simulink model "AcasXuClosedLoop_all"  (Simulink 10.0)
settings: Solver=VariableStepAuto  StartTime=0.0  StopTime=20  RelTol=1e-3
libraries: neural x14040, simulink x2
(part 1 of 534)
settings Model: Creator=manzand  LastModifiedBy=manzand
settings DataIOCC: SaveFormat=Dataset  ReturnWorkspaceOutputs=on
settings OptimizationCC: LifeSpan=auto
settings DebuggingCC: UnderspecifiedInitializationDetection=Simplified  MergeDetectMultiDrivingBlocksExec=error  SFUnexpectedBacktrackingDiag=error  SFNoUnconditionalDefaultTransitionDiag=error  SFExecutionAtInitializationDiag=warning  ArithmeticOperatorsInVariantConditions=error
settings HardwareCC: ProdBitPerPointer=64  ProdBitPerSizeT=64  ProdBitPerPtrDiffT=64  ProdLargestAtomicFloat=Float  ProdIntDivRoundTo=Zero  ProdEndianess=LittleEndian  ProdWordSize=64  ProdHWDeviceType=Intel->x86-64 (Windows64)
settings ModelReferenceCC: PropagateSignalLabelsOutOfModel=on
settings SFSimCC: SimUseLocalCustomCode=on  CustomCodeUndefinedFunction=FilterOut
settings RTWCC: RTWUseLocalCustomCode=on
settings CodeAppCC: StateflowObjectComments=off
settings GRTTargetCC: TargetLangStandard=C99 (ISO)  CombineOutputUpdateFcns=on  GRTInterface=off
settings ConfigComp: CovMetricSettings=dwe  CovHtmlReporting=off  CovSaveCumulativeToWorkspaceVar=off  CovSaveSingleToWorkspaceVar=off

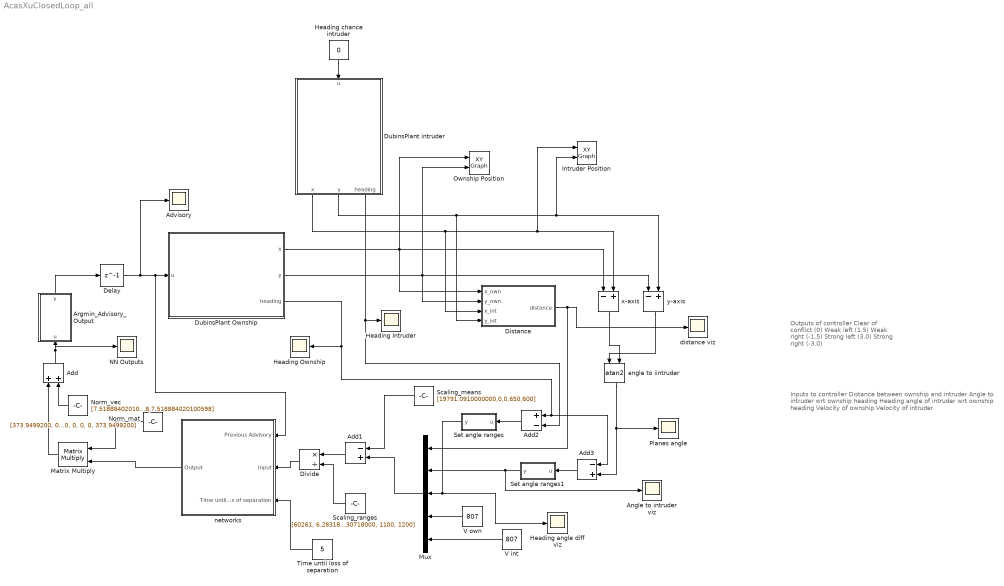
== system AcasXuClosedLoop_all ==
  note: Outputs of controller
      | Clear of conflict (0)
      | Weak left (1.5)
      | Weak right (-1.5)
      | Strong left (3.0)
      | Strong right (-3.0)
  note: Inputs to controller
      | Distance between ownship and intruder
      | Angle to intruder wrt ownship heading
      | Heading angle of intruder wrt ownship heading
      | Velocity of ownship
      | Velocity of intruder
  Add : Sum  Priority=20
  Add1 : Sum  Priority=14  Inputs=-+
  Add2 : Sum  Priority=9  Inputs=+-
  Add3 : Sum  Priority=9  Inputs=-+
  Advisory : Scope
  Angle to intruder viz : Scope
  Argmin_Advisory_Output : MATLAB Function  Priority=21  TreatAsAtomicUnit=on
  Delay : Delay  Priority=1  DelayLength=1  InitialCondition=0
  Distance : MATLAB Function  Priority=5  TreatAsAtomicUnit=on
  Divide : Product  Priority=16  Inputs=*/
  DubinsPlant Ownship : Stateflow chart  Priority=2  BlockKeywords=__PRM__SERIALIZED__DATA__:Stateflow Chart,Stateflow Diagram  TreatAsAtomicUnit=on
  DubinsPlant intruder : Stateflow chart  Priority=4  BlockKeywords=__PRM__SERIALIZED__DATA__:Stateflow Chart,Stateflow Diagram  TreatAsAtomicUnit=on
  Heading Intruder : Scope
  Heading Ownship : Scope
  Heading angle diff viz : Scope
  "Heading chance intruder " : Constant  Priority=3  Value=0
  Intruder Position : simulink/Sinks/XY Graph  xmin=-100  xmax=100  ymin=10000  ymax=14000  st=-1
  Matrix Multiply : Product  Priority=19  Multiplication=Matrix(*)
  Mux : Mux  Inputs=5
  NN Outputs : Scope
  Norm_mat : Constant  Priority=18  Value=|  VectorParams1D=off
      | [373.9499200, 0, 0, 0, 0; 0, 373.9499200, 0, 0, 0;
      | 0, 0, 373.9499200, 0, 0;
      | 0, 0, 0, 373.9499200, 0;
      | 0, 0, 0, 0, 373.9499200]
  "Norm_vec " : Constant  Value=[7.518884020100598, 7.518884020100598,7.518884020100598,7.518884020100598,7.518884020100598]
  Ownship Position : simulink/Sinks/XY Graph  xmin=-5000  xmax=600  ymin=1  ymax=23000  st=-1
  Planes angle : Scope
  Scaling_means : Constant  Priority=13  Value=[19791.0910000000,0,0,650,600]
  Scaling_ranges : Constant  Priority=15  Value=[60261, 6.28318530718000, 6.28318530718000, 1100, 1200]  VectorParams1D=off
  Set angle ranges : MATLAB Function  TreatAsAtomicUnit=on
  Set angle ranges1 : MATLAB Function  TreatAsAtomicUnit=on
  Time until loss of separation : Constant  Priority=10  Value=5
  V int : Constant  Priority=11  Value=807
  V own : Constant  Priority=10  Value=807
  angle to iintruder : Trigonometry  Priority=8  Operator=atan2  OutputSignalType=real
  distance viz : Scope
  networks : Subsystem
  x-axis : Sum  Priority=6  Inputs=-+
  y-axis : Sum  Priority=7  Inputs=-+
  wires:
  "Heading chance intruder "/1 -> DubinsPlant intruder/1(u)
  Argmin_Advisory_Output/1(y) -> Delay/1
  Scaling_ranges/1 -> Divide/2
  V int/1 -> Mux/5
  Add2/1 -> Set angle ranges/1(u)
  y-axis/1 -> angle to iintruder/1
  Add3/1 -> Set angle ranges1/1(u)
  Divide/1 -> networks/2(Input)
  Add1/1 -> Divide/1
  V own/1 -> Mux/4
  x-axis/1 -> angle to iintruder/2
  "Norm_vec "/1 -> Add/2
  Add/1 -> NN Outputs/1, Argmin_Advisory_Output/1(u)
  angle to iintruder/1 -> Planes angle/1, Add3/2
  Norm_mat/1 -> Matrix Multiply/1
  Scaling_means/1 -> Add1/1
  Distance/1(distance) -> Mux/1, distance viz/1
  DubinsPlant Ownship/3(heading) -> Heading Ownship/1, Add3/1, Add2/1
  DubinsPlant Ownship/1(x) -> Distance/1(x_own), x-axis/1, Ownship Position/1
  DubinsPlant intruder/2(y) -> Distance/4(y_int), y-axis/2, Intruder Position/2
  DubinsPlant intruder/1(x) -> Distance/3(x_int), x-axis/2, Intruder Position/1
  DubinsPlant intruder/3(heading) -> Heading Intruder/1, Add2/2
  DubinsPlant Ownship/2(y) -> Distance/2(y_own), y-axis/1, Ownship Position/2
  Delay/1 -> networks/1(Previous Advisory), DubinsPlant Ownship/1(u), Advisory/1
  networks/1(Output) -> Matrix Multiply/2
  Mux/1 -> Add1/2
  Matrix Multiply/1 -> Add/1
  Time until loss of separation/1 -> networks/3("Time until  loss of separation ")
  Set angle ranges/1(y) -> Mux/3, Heading angle diff viz/1
  Set angle ranges1/1(y) -> Angle to intruder viz/1, Mux/2

== system AcasXuClosedLoop_all/networks ==
  Previous Advisory : Inport
  Input : Inport  Port=2
  "Time until  loss of separation " : Inport  Port=3
  If : If  IfExpression=u1 == 0  ElseIfExpressions=u1 == 1, u1 == 5, u1 == 10, u1 == 20, u1 == 40, u1 == 60, u1 == 80, u1 == 100  ShowElse=off
  If Action Subsystem : Subsystem  TreatAsAtomicUnit=on
  If Action Subsystem1 : Subsystem  TreatAsAtomicUnit=on
  If Action Subsystem2 : Subsystem  TreatAsAtomicUnit=on
  If Action Subsystem3 : Subsystem  TreatAsAtomicUnit=on
  If Action Subsystem4 : Subsystem  TreatAsAtomicUnit=on
  If Action Subsystem5 : Subsystem  TreatAsAtomicUnit=on
  If Action Subsystem6 : Subsystem  TreatAsAtomicUnit=on
  If Action Subsystem7 : Subsystem  TreatAsAtomicUnit=on
  If Action Subsystem8 : Subsystem  TreatAsAtomicUnit=on
  Input{1..8} (8 alike) : InportShadow  Port=2
  Merge : Merge  Inputs=9
  Networks_m_1 : Subsystem
  Networks_m_2 : Subsystem
  Networks_m_3 : Subsystem
  Networks_m_4 : Subsystem
  Networks_m_5 : Subsystem
  Networks_m_6 : Subsystem
  Networks_m_7 : Subsystem
  Networks_m_8 : Subsystem
  Networks_m_9 : Subsystem
  Previous Advisory{1..8} (8 alike) : InportShadow
  Output : Outport
  wires:
  Merge/1 -> Output/1
  Networks_m_1/1(Out1) -> If Action Subsystem/1(In1)
  If Action Subsystem/1(Out1) -> Merge/1
  If Action Subsystem1/1(Out1) -> Merge/2
  Networks_m_2/1(Out1) -> If Action Subsystem1/1(In1)
  Networks_m_3/1(Out1) -> If Action Subsystem2/1(In1)
  If Action Subsystem2/1(Out1) -> Merge/3
  Networks_m_4/1(Out1) -> If Action Subsystem3/1(In1)
  If Action Subsystem3/1(Out1) -> Merge/4
  Networks_m_5/1(Out1) -> If Action Subsystem4/1(In1)
  If Action Subsystem4/1(Out1) -> Merge/5
  Networks_m_6/1(Out1) -> If Action Subsystem5/1(In1)
  If Action Subsystem5/1(Out1) -> Merge/6
  Networks_m_7/1(Out1) -> If Action Subsystem6/1(In1)
  If Action Subsystem6/1(Out1) -> Merge/7
  Networks_m_8/1(Out1) -> If Action Subsystem7/1(In1)
  If Action Subsystem7/1(Out1) -> Merge/8
  Networks_m_9/1(Out1) -> If Action Subsystem8/1(In1)
  If Action Subsystem8/1(Out1) -> Merge/9
  "Time until  loss of separation "/1 -> If/1
  Previous Advisory/1 -> Networks_m_1/2(Prev. advisory)
  If/1 -> If Action Subsystem/Action
  Input/1 -> Networks_m_1/1(Input)
  If/2 -> If Action Subsystem1/Action
  If/3 -> If Action Subsystem2/Action
  If/4 -> If Action Subsystem3/Action
  If/5 -> If Action Subsystem4/Action
  If/6 -> If Action Subsystem5/Action
  If/7 -> If Action Subsystem6/Action
  If/8 -> If Action Subsystem7/Action
  If/9 -> If Action Subsystem8/Action
  Input1/1 -> Networks_m_2/1(Input)
  Input2/1 -> Networks_m_3/1(Input)
  Input3/1 -> Networks_m_4/1(Input)
  Input4/1 -> Networks_m_5/1(Input)
  Input5/1 -> Networks_m_6/1(Input)
  Input6/1 -> Networks_m_7/1(Input)
  Input7/1 -> Networks_m_8/1(Input)
  Input8/1 -> Networks_m_9/1(Input)
  Previous Advisory1/1 -> Networks_m_2/2(Prev. advisory)
  Previous Advisory2/1 -> Networks_m_3/2(Prev. advisory)
  Previous Advisory3/1 -> Networks_m_4/2(Prev. advisory)
  Previous Advisory4/1 -> Networks_m_5/2(Prev. advisory)
  Previous Advisory5/1 -> Networks_m_6/2(Prev. advisory)
  Previous Advisory6/1 -> Networks_m_7/2(Prev. advisory)
  Previous Advisory7/1 -> Networks_m_8/2(Prev. advisory)
  Previous Advisory8/1 -> Networks_m_9/2(Prev. advisory)

== system AcasXuClosedLoop_all/networks/If Action Subsystem ==
  In1 : Inport
  Action Port : ActionPort  ActionPortLabel=if(u1 == 0)
  Out1 : Outport
  wires:
  In1/1 -> Out1/1

== system AcasXuClosedLoop_all/networks/If Action Subsystem1 ==
  In1 : Inport
  Action Port : ActionPort  ActionPortLabel=elseif(u1 == 1)
  Out1 : Outport
  wires:
  In1/1 -> Out1/1

== system AcasXuClosedLoop_all/networks/If Action Subsystem2 ==
  In1 : Inport
  Action Port : ActionPort  ActionPortLabel=elseif( u1 == 5)
  Out1 : Outport
  wires:
  In1/1 -> Out1/1

== system AcasXuClosedLoop_all/networks/If Action Subsystem3 ==
  In1 : Inport
  Action Port : ActionPort  ActionPortLabel=elseif( u1 == 10)
  Out1 : Outport
  wires:
  In1/1 -> Out1/1

== system AcasXuClosedLoop_all/networks/If Action Subsystem4 ==
  In1 : Inport
  Action Port : ActionPort  ActionPortLabel=elseif( u1 == 20)
  Out1 : Outport
  wires:
  In1/1 -> Out1/1

== system AcasXuClosedLoop_all/networks/If Action Subsystem5 ==
  In1 : Inport
  Action Port : ActionPort  ActionPortLabel=elseif( u1 == 40)
  Out1 : Outport
  wires:
  In1/1 -> Out1/1

== system AcasXuClosedLoop_all/networks/If Action Subsystem6 ==
  In1 : Inport
  Action Port : ActionPort  ActionPortLabel=elseif( u1 == 60)
  Out1 : Outport
  wires:
  In1/1 -> Out1/1

== system AcasXuClosedLoop_all/networks/If Action Subsystem7 ==
  In1 : Inport
  Action Port : ActionPort  ActionPortLabel=elseif( u1 == 80)
  Out1 : Outport
  wires:
  In1/1 -> Out1/1

== system AcasXuClosedLoop_all/networks/If Action Subsystem8 ==
  In1 : Inport
  Action Port : ActionPort  ActionPortLabel=elseif( u1 == 100)
  Out1 : Outport
  wires:
  In1/1 -> Out1/1

== system AcasXuClosedLoop_all/networks/Networks_m_1 ==
  Input : Inport
  Prev. advisory : Inport  Port=2
  AcasXu_1_1 : Subsystem
      mask help: Feed-Forward Neural Network
      mask Display: e = 0.06;c1 = [120 150 230]/255;c2 = ([120 150 230]+0)/383;c3 = ([120 150 230]+128)/383;patch([0 1 1 0],[0 0 1 1],c1);patch([0 1 1 1-e 1-e 0+e],[0 0 1 1-e 0+e 0+e],c2);patch([0 0 1 1-e 0+e 0+e],[0 1 1 1-e 1-e 0+e],c3);plot([0 1 1 0 0],[0 0 1 1 0]);fprintf('NNET');port_label('input',1,' Input');port_label('output',1,'Output ');
  AcasXu_2_1 : Subsystem
      mask help: Feed-Forward Neural Network
      mask Display: e = 0.06;c1 = [120 150 230]/255;c2 = ([120 150 230]+0)/383;c3 = ([120 150 230]+128)/383;patch([0 1 1 0],[0 0 1 1],c1);patch([0 1 1 1-e 1-e 0+e],[0 0 1 1-e 0+e 0+e],c2);patch([0 0 1 1-e 0+e 0+e],[0 1 1 1-e 1-e 0+e],c3);plot([0 1 1 0 0],[0 0 1 1 0]);fprintf('NNET');port_label('input',1,' Input');port_label('output',1,'Output ');
  AcasXu_3_1 : Subsystem
      mask help: Feed-Forward Neural Network
      mask Display: e = 0.06;c1 = [120 150 230]/255;c2 = ([120 150 230]+0)/383;c3 = ([120 150 230]+128)/383;patch([0 1 1 0],[0 0 1 1],c1);patch([0 1 1 1-e 1-e 0+e],[0 0 1 1-e 0+e 0+e],c2);patch([0 0 1 1-e 0+e 0+e],[0 1 1 1-e 1-e 0+e],c3);plot([0 1 1 0 0],[0 0 1 1 0]);fprintf('NNET');port_label('input',1,' Input');port_label('output',1,'Output ');
  AcasXu_4_1 : Subsystem
      mask help: Feed-Forward Neural Network
      mask Display: e = 0.06;c1 = [120 150 230]/255;c2 = ([120 150 230]+0)/383;c3 = ([120 150 230]+128)/383;patch([0 1 1 0],[0 0 1 1],c1);patch([0 1 1 1-e 1-e 0+e],[0 0 1 1-e 0+e 0+e],c2);patch([0 0 1 1-e 0+e 0+e],[0 1 1 1-e 1-e 0+e],c3);plot([0 1 1 0 0],[0 0 1 1 0]);fprintf('NNET');port_label('input',1,' Input');port_label('output',1,'Output ');
  AcasXu_5_1 : Subsystem
      mask help: Feed-Forward Neural Network
      mask Display: e = 0.06;c1 = [120 150 230]/255;c2 = ([120 150 230]+0)/383;c3 = ([120 150 230]+128)/383;patch([0 1 1 0],[0 0 1 1],c1);patch([0 1 1 1-e 1-e 0+e],[0 0 1 1-e 0+e 0+e],c2);patch([0 0 1 1-e 0+e 0+e],[0 1 1 1-e 1-e 0+e],c3);plot([0 1 1 0 0],[0 0 1 1 0]);fprintf('NNET');port_label('input',1,' Input');port_label('output',1,'Output ');
  If : If  IfExpression=u1 == 0  ElseIfExpressions=u1 == deg2rad(1.5), u1 == deg2rad(-1.5), u1 == deg2rad(3.0), u1 == deg2rad(-3.0)  ShowElse=off
  If Action Subsystem : Subsystem  TreatAsAtomicUnit=on
  If Action Subsystem1 : Subsystem  TreatAsAtomicUnit=on
  If Action Subsystem2 : Subsystem  TreatAsAtomicUnit=on
  If Action Subsystem3 : Subsystem  TreatAsAtomicUnit=on
  If Action Subsystem4 : Subsystem  TreatAsAtomicUnit=on
  Merge : Merge  Inputs=5
  Out1 : Outport
  wires:
  If Action Subsystem2/1(Out1) -> Merge/3
  If/1 -> If Action Subsystem/Action
  If/3 -> If Action Subsystem2/Action
  If Action Subsystem4/1(Out1) -> Merge/5
  Input/1 -> AcasXu_5_1/1(Input), AcasXu_4_1/1(Input), AcasXu_3_1/1(Input), AcasXu_1_1/1(Input), AcasXu_2_1/1(Input)
  If/2 -> If Action Subsystem1/Action
  If Action Subsystem1/1(Out1) -> Merge/2
  AcasXu_1_1/1(Output) -> If Action Subsystem/1(In1)
  Merge/1 -> Out1/1
  Prev. advisory/1 -> If/1
  If Action Subsystem3/1(Out1) -> Merge/4
  If/4 -> If Action Subsystem3/Action
  If/5 -> If Action Subsystem4/Action
  If Action Subsystem/1(Out1) -> Merge/1
  AcasXu_2_1/1(Output) -> If Action Subsystem1/1(In1)
  AcasXu_3_1/1(Output) -> If Action Subsystem2/1(In1)
  AcasXu_4_1/1(Output) -> If Action Subsystem3/1(In1)
  AcasXu_5_1/1(Output) -> If Action Subsystem4/1(In1)

== system AcasXuClosedLoop_all/networks/Networks_m_2 ==
  Input : Inport
  Prev. advisory : Inport  Port=2
  AcasXu_1_2 : Subsystem
      mask help: Feed-Forward Neural Network
      mask Display: e = 0.06;c1 = [120 150 230]/255;c2 = ([120 150 230]+0)/383;c3 = ([120 150 230]+128)/383;patch([0 1 1 0],[0 0 1 1],c1);patch([0 1 1 1-e 1-e 0+e],[0 0 1 1-e 0+e 0+e],c2);patch([0 0 1 1-e 0+e 0+e],[0 1 1 1-e 1-e 0+e],c3);plot([0 1 1 0 0],[0 0 1 1 0]);fprintf('NNET');port_label('input',1,' Input');port_label('output',1,'Output ');
  AcasXu_2_2 : Subsystem
      mask help: Feed-Forward Neural Network
      mask Display: e = 0.06;c1 = [120 150 230]/255;c2 = ([120 150 230]+0)/383;c3 = ([120 150 230]+128)/383;patch([0 1 1 0],[0 0 1 1],c1);patch([0 1 1 1-e 1-e 0+e],[0 0 1 1-e 0+e 0+e],c2);patch([0 0 1 1-e 0+e 0+e],[0 1 1 1-e 1-e 0+e],c3);plot([0 1 1 0 0],[0 0 1 1 0]);fprintf('NNET');port_label('input',1,' Input');port_label('output',1,'Output ');
  AcasXu_3_2 : Subsystem
      mask help: Feed-Forward Neural Network
      mask Display: e = 0.06;c1 = [120 150 230]/255;c2 = ([120 150 230]+0)/383;c3 = ([120 150 230]+128)/383;patch([0 1 1 0],[0 0 1 1],c1);patch([0 1 1 1-e 1-e 0+e],[0 0 1 1-e 0+e 0+e],c2);patch([0 0 1 1-e 0+e 0+e],[0 1 1 1-e 1-e 0+e],c3);plot([0 1 1 0 0],[0 0 1 1 0]);fprintf('NNET');port_label('input',1,' Input');port_label('output',1,'Output ');
  AcasXu_4_2 : Subsystem
      mask help: Feed-Forward Neural Network
      mask Display: e = 0.06;c1 = [120 150 230]/255;c2 = ([120 150 230]+0)/383;c3 = ([120 150 230]+128)/383;patch([0 1 1 0],[0 0 1 1],c1);patch([0 1 1 1-e 1-e 0+e],[0 0 1 1-e 0+e 0+e],c2);patch([0 0 1 1-e 0+e 0+e],[0 1 1 1-e 1-e 0+e],c3);plot([0 1 1 0 0],[0 0 1 1 0]);fprintf('NNET');port_label('input',1,' Input');port_label('output',1,'Output ');
  AcasXu_5_2 : Subsystem
      mask help: Feed-Forward Neural Network
      mask Display: e = 0.06;c1 = [120 150 230]/255;c2 = ([120 150 230]+0)/383;c3 = ([120 150 230]+128)/383;patch([0 1 1 0],[0 0 1 1],c1);patch([0 1 1 1-e 1-e 0+e],[0 0 1 1-e 0+e 0+e],c2);patch([0 0 1 1-e 0+e 0+e],[0 1 1 1-e 1-e 0+e],c3);plot([0 1 1 0 0],[0 0 1 1 0]);fprintf('NNET');port_label('input',1,' Input');port_label('output',1,'Output ');
  If : If  IfExpression=u1 == 0  ElseIfExpressions=u1 == deg2rad(1.5), u1 == deg2rad(-1.5), u1 == deg2rad(3.0), u1 == deg2rad(-3.0)  ShowElse=off
  If Action Subsystem : Subsystem  TreatAsAtomicUnit=on
  If Action Subsystem1 : Subsystem  TreatAsAtomicUnit=on
  If Action Subsystem2 : Subsystem  TreatAsAtomicUnit=on
  If Action Subsystem3 : Subsystem  TreatAsAtomicUnit=on
  If Action Subsystem4 : Subsystem  TreatAsAtomicUnit=on
  Merge : Merge  Inputs=5
  Out1 : Outport
  wires:
  AcasXu_5_2/1(Output) -> If Action Subsystem4/1(In1)
  AcasXu_4_2/1(Output) -> If Action Subsystem3/1(In1)
  AcasXu_3_2/1(Output) -> If Action Subsystem2/1(In1)
  AcasXu_2_2/1(Output) -> If Action Subsystem1/1(In1)
  If Action Subsystem/1(Out1) -> Merge/1
  If/5 -> If Action Subsystem4/Action
  If/4 -> If Action Subsystem3/Action
  If Action Subsystem3/1(Out1) -> Merge/4
  Prev. advisory/1 -> If/1
  Merge/1 -> Out1/1
  AcasXu_1_2/1(Output) -> If Action Subsystem/1(In1)
  If Action Subsystem1/1(Out1) -> Merge/2
  If/2 -> If Action Subsystem1/Action
  Input/1 -> AcasXu_2_2/1(Input), AcasXu_1_2/1(Input), AcasXu_3_2/1(Input), AcasXu_5_2/1(Input), AcasXu_4_2/1(Input)
  If Action Subsystem4/1(Out1) -> Merge/5
  If/3 -> If Action Subsystem2/Action
  If/1 -> If Action Subsystem/Action
  If Action Subsystem2/1(Out1) -> Merge/3

== system AcasXuClosedLoop_all/networks/Networks_m_3 ==
  Input : Inport
  Prev. advisory : Inport  Port=2
  AcasXu_1_3 : Subsystem
      mask help: Feed-Forward Neural Network
      mask Display: e = 0.06;c1 = [120 150 230]/255;c2 = ([120 150 230]+0)/383;c3 = ([120 150 230]+128)/383;patch([0 1 1 0],[0 0 1 1],c1);patch([0 1 1 1-e 1-e 0+e],[0 0 1 1-e 0+e 0+e],c2);patch([0 0 1 1-e 0+e 0+e],[0 1 1 1-e 1-e 0+e],c3);plot([0 1 1 0 0],[0 0 1 1 0]);fprintf('NNET');port_label('input',1,' Input');port_label('output',1,'Output ');
  AcasXu_2_3 : Subsystem
      mask help: Feed-Forward Neural Network
      mask Display: e = 0.06;c1 = [120 150 230]/255;c2 = ([120 150 230]+0)/383;c3 = ([120 150 230]+128)/383;patch([0 1 1 0],[0 0 1 1],c1);patch([0 1 1 1-e 1-e 0+e],[0 0 1 1-e 0+e 0+e],c2);patch([0 0 1 1-e 0+e 0+e],[0 1 1 1-e 1-e 0+e],c3);plot([0 1 1 0 0],[0 0 1 1 0]);fprintf('NNET');port_label('input',1,' Input');port_label('output',1,'Output ');
  AcasXu_3_3 : Subsystem
      mask help: Feed-Forward Neural Network
      mask Display: e = 0.06;c1 = [120 150 230]/255;c2 = ([120 150 230]+0)/383;c3 = ([120 150 230]+128)/383;patch([0 1 1 0],[0 0 1 1],c1);patch([0 1 1 1-e 1-e 0+e],[0 0 1 1-e 0+e 0+e],c2);patch([0 0 1 1-e 0+e 0+e],[0 1 1 1-e 1-e 0+e],c3);plot([0 1 1 0 0],[0 0 1 1 0]);fprintf('NNET');port_label('input',1,' Input');port_label('output',1,'Output ');
  AcasXu_4_3 : Subsystem
      mask help: Feed-Forward Neural Network
      mask Display: e = 0.06;c1 = [120 150 230]/255;c2 = ([120 150 230]+0)/383;c3 = ([120 150 230]+128)/383;patch([0 1 1 0],[0 0 1 1],c1);patch([0 1 1 1-e 1-e 0+e],[0 0 1 1-e 0+e 0+e],c2);patch([0 0 1 1-e 0+e 0+e],[0 1 1 1-e 1-e 0+e],c3);plot([0 1 1 0 0],[0 0 1 1 0]);fprintf('NNET');port_label('input',1,' Input');port_label('output',1,'Output ');
  AcasXu_5_3 : Subsystem
      mask help: Feed-Forward Neural Network
      mask Display: e = 0.06;c1 = [120 150 230]/255;c2 = ([120 150 230]+0)/383;c3 = ([120 150 230]+128)/383;patch([0 1 1 0],[0 0 1 1],c1);patch([0 1 1 1-e 1-e 0+e],[0 0 1 1-e 0+e 0+e],c2);patch([0 0 1 1-e 0+e 0+e],[0 1 1 1-e 1-e 0+e],c3);plot([0 1 1 0 0],[0 0 1 1 0]);fprintf('NNET');port_label('input',1,' Input');port_label('output',1,'Output ');
  If : If  IfExpression=u1 == 0  ElseIfExpressions=u1 == deg2rad(1.5), u1 == deg2rad(-1.5), u1 == deg2rad(3.0), u1 == deg2rad(-3.0)  ShowElse=off
  If Action Subsystem : Subsystem  TreatAsAtomicUnit=on
  If Action Subsystem1 : Subsystem  TreatAsAtomicUnit=on
  If Action Subsystem2 : Subsystem  TreatAsAtomicUnit=on
  If Action Subsystem3 : Subsystem  TreatAsAtomicUnit=on
  If Action Subsystem4 : Subsystem  TreatAsAtomicUnit=on
  Merge : Merge  Inputs=5
  Out1 : Outport
  wires:
  If Action Subsystem2/1(Out1) -> Merge/3
  If/1 -> If Action Subsystem/Action
  If/3 -> If Action Subsystem2/Action
  If Action Subsystem4/1(Out1) -> Merge/5
  Input/1 -> AcasXu_3_3/1(Input), AcasXu_5_3/1(Input), AcasXu_4_3/1(Input), AcasXu_2_3/1(Input), AcasXu_1_3/1(Input)
  If/2 -> If Action Subsystem1/Action
  If Action Subsystem1/1(Out1) -> Merge/2
  AcasXu_1_3/1(Output) -> If Action Subsystem/1(In1)
  Merge/1 -> Out1/1
  Prev. advisory/1 -> If/1
  If Action Subsystem3/1(Out1) -> Merge/4
  If/4 -> If Action Subsystem3/Action
  If/5 -> If Action Subsystem4/Action
  If Action Subsystem/1(Out1) -> Merge/1
  AcasXu_2_3/1(Output) -> If Action Subsystem1/1(In1)
  AcasXu_3_3/1(Output) -> If Action Subsystem2/1(In1)
  AcasXu_4_3/1(Output) -> If Action Subsystem3/1(In1)
  AcasXu_5_3/1(Output) -> If Action Subsystem4/1(In1)

== system AcasXuClosedLoop_all/networks/Networks_m_4 ==
  Input : Inport
  Prev. advisory : Inport  Port=2
  AcasXu_1_4 : Subsystem
      mask help: Feed-Forward Neural Network
      mask Display: e = 0.06;c1 = [120 150 230]/255;c2 = ([120 150 230]+0)/383;c3 = ([120 150 230]+128)/383;patch([0 1 1 0],[0 0 1 1],c1);patch([0 1 1 1-e 1-e 0+e],[0 0 1 1-e 0+e 0+e],c2);patch([0 0 1 1-e 0+e 0+e],[0 1 1 1-e 1-e 0+e],c3);plot([0 1 1 0 0],[0 0 1 1 0]);fprintf('NNET');port_label('input',1,' Input');port_label('output',1,'Output ');
  AcasXu_2_4 : Subsystem
      mask help: Feed-Forward Neural Network
      mask Display: e = 0.06;c1 = [120 150 230]/255;c2 = ([120 150 230]+0)/383;c3 = ([120 150 230]+128)/383;patch([0 1 1 0],[0 0 1 1],c1);patch([0 1 1 1-e 1-e 0+e],[0 0 1 1-e 0+e 0+e],c2);patch([0 0 1 1-e 0+e 0+e],[0 1 1 1-e 1-e 0+e],c3);plot([0 1 1 0 0],[0 0 1 1 0]);fprintf('NNET');port_label('input',1,' Input');port_label('output',1,'Output ');
  AcasXu_3_4 : Subsystem
      mask help: Feed-Forward Neural Network
      mask Display: e = 0.06;c1 = [120 150 230]/255;c2 = ([120 150 230]+0)/383;c3 = ([120 150 230]+128)/383;patch([0 1 1 0],[0 0 1 1],c1);patch([0 1 1 1-e 1-e 0+e],[0 0 1 1-e 0+e 0+e],c2);patch([0 0 1 1-e 0+e 0+e],[0 1 1 1-e 1-e 0+e],c3);plot([0 1 1 0 0],[0 0 1 1 0]);fprintf('NNET');port_label('input',1,' Input');port_label('output',1,'Output ');
  AcasXu_4_4 : Subsystem
      mask help: Feed-Forward Neural Network
      mask Display: e = 0.06;c1 = [120 150 230]/255;c2 = ([120 150 230]+0)/383;c3 = ([120 150 230]+128)/383;patch([0 1 1 0],[0 0 1 1],c1);patch([0 1 1 1-e 1-e 0+e],[0 0 1 1-e 0+e 0+e],c2);patch([0 0 1 1-e 0+e 0+e],[0 1 1 1-e 1-e 0+e],c3);plot([0 1 1 0 0],[0 0 1 1 0]);fprintf('NNET');port_label('input',1,' Input');port_label('output',1,'Output ');
  AcasXu_5_4 : Subsystem
      mask help: Feed-Forward Neural Network
      mask Display: e = 0.06;c1 = [120 150 230]/255;c2 = ([120 150 230]+0)/383;c3 = ([120 150 230]+128)/383;patch([0 1 1 0],[0 0 1 1],c1);patch([0 1 1 1-e 1-e 0+e],[0 0 1 1-e 0+e 0+e],c2);patch([0 0 1 1-e 0+e 0+e],[0 1 1 1-e 1-e 0+e],c3);plot([0 1 1 0 0],[0 0 1 1 0]);fprintf('NNET');port_label('input',1,' Input');port_label('output',1,'Output ');
  If : If  IfExpression=u1 == 0  ElseIfExpressions=u1 == deg2rad(1.5), u1 == deg2rad(-1.5), u1 == deg2rad(3.0), u1 == deg2rad(-3.0)  ShowElse=off
  If Action Subsystem : Subsystem  TreatAsAtomicUnit=on
  If Action Subsystem1 : Subsystem  TreatAsAtomicUnit=on
  If Action Subsystem2 : Subsystem  TreatAsAtomicUnit=on
  If Action Subsystem3 : Subsystem  TreatAsAtomicUnit=on
  If Action Subsystem4 : Subsystem  TreatAsAtomicUnit=on
  Merge : Merge  Inputs=5
  Out1 : Outport
  wires:
  AcasXu_5_4/1(Output) -> If Action Subsystem4/1(In1)
  AcasXu_4_4/1(Output) -> If Action Subsystem3/1(In1)
  AcasXu_3_4/1(Output) -> If Action Subsystem2/1(In1)
  AcasXu_2_4/1(Output) -> If Action Subsystem1/1(In1)
  If Action Subsystem/1(Out1) -> Merge/1
  If/5 -> If Action Subsystem4/Action
  If/4 -> If Action Subsystem3/Action
  If Action Subsystem3/1(Out1) -> Merge/4
  Prev. advisory/1 -> If/1
  Merge/1 -> Out1/1
  AcasXu_1_4/1(Output) -> If Action Subsystem/1(In1)
  If Action Subsystem1/1(Out1) -> Merge/2
  If/2 -> If Action Subsystem1/Action
  Input/1 -> AcasXu_2_4/1(Input), AcasXu_1_4/1(Input), AcasXu_3_4/1(Input), AcasXu_5_4/1(Input), AcasXu_4_4/1(Input)
  If Action Subsystem4/1(Out1) -> Merge/5
  If/3 -> If Action Subsystem2/Action